simulink model "controller_observer_AllAgents_v5"  (Simulink 8.4)
settings: Solver=ode1  StartTime=0.0  StopTime=inf  FixedStep=60*1  RelTol=1e-3
values from neighbouring files (symbols this model uses):
  Cpw = 4180    [readepJSONout.m]
  m_max = 3    [DesignParameters.m]
  mc_MAX = 2    [DesignParameters.m]
  Tamb_additive_Sensor_fault_time = 60*60*24*(Tamb_day_ofthe_add_fault + Tamb_time_ofthe_add_fault)    (= 129600)    [faults_setting.m]
  Tamb_additive_Sensor_fault_value = 10    [faults_setting.m]
  Tamb_mult_Sensor_fault_time = 60*60*24*(Tamb_day_ofthe_mult_fault + Tamb_time_ofthe_mult_fault)    (= 129600)    [faults_setting.m]
  Tamb_mult_Sensor_fault_value = 1.1    [faults_setting.m]
  Ts = 3600/dat.Timestep.Timestep_0x20_1.number_of_timesteps_per_hour    [readepJSONout.m]
  Tst_C_additive_Sensor_fault_time = 60*60*24*(Tst_C_day_ofthe_add_fault + Tst_C_time_ofthe_add_fault)    (= 129600)    [faults_setting.m]
  Tst_C_additive_Sensor_fault_value = 10    [faults_setting.m]
  Tst_C_mult_Sensor_fault_time = 60*60*24*(Tst_C_day_ofthe_mult_fault + Tst_C_time_ofthe_mult_fault)    (= 129600)    [faults_setting.m]
  Tst_C_mult_Sensor_fault_value = 1.1    [faults_setting.m]
  Tst_H_additive_Sensor_fault_time = 60*60*24*(Tst_H_day_ofthe_add_fault + Tst_H_time_ofthe_add_fault)    (= 129600)    [faults_setting.m]
  Tst_H_additive_Sensor_fault_value = 10    [faults_setting.m]
  Tst_H_mult_Sensor_fault_time = 60*60*24*(Tst_H_day_ofthe_mult_fault + Tst_H_time_ofthe_mult_fault)    (= 129600)    [faults_setting.m]
  Tst_H_mult_Sensor_fault_value = 1.1    [faults_setting.m]
undefined symbols (not defined in the file or in its folder): building, COP, Ehp_max, fsa_E, fsa_ET, fsa_N, fsa_NT, fsa_W, fsa_WT, h_E, h_ET, h_N, h_NT, h_W, h_WT, K_NtoNT, K_NTtoN, Ke_E, Ke_ET, Ke_N, Ke_NT, Ke_WT, Km_E, Km_ET, Km_N, Km_NT, Km_W, Km_WT, Kout_N, Kout_NT, Kout_W, Kout_WT, Kst, Ksta, Kstcij, KstRef, Kw_E, Kw_ET, Kw_N, Kw_NT, Kw_W, Kw_WT
libraries: simulink x61
(part 36 of 36)

== system controller_observer_AllAgents_v5/Controller/MultiClass1Pod3_Agent/Threshold_hcoil/counter ==
  1 : Constant  SampleTime=Ts
  Delay2 : Delay  DelayLength=1  InitialCondition=0  SampleTime=Ts
  Sum : Sum  Inputs=|++
  Out1 : Outport
  Out2 : Outport  Port=2
  wires:
  1/1 -> Sum/2
  Delay2/1 -> Out1/1, Sum/1
  Sum/1 -> Out2/1, Delay2/1

== system controller_observer_AllAgents_v5/Controller/MultiClass1Pod3_Agent/Threshold_sa/If Action Subsystem2 ==
  In1 : Inport
  Action Port : ActionPort
  Out1 : Outport
  wires:
  In1/1 -> Out1/1

== system controller_observer_AllAgents_v5/Controller/MultiClass1Pod3_Agent/Threshold_sa/If Action Subsystem3 ==
  In1 : Inport
  Action Port : ActionPort
  Out1 : Outport
  wires:
  In1/1 -> Out1/1

== system controller_observer_AllAgents_v5/Controller/MultiClass1Pod3_Agent/Threshold_sa/counter ==
  1 : Constant  SampleTime=Ts
  Delay2 : Delay  DelayLength=1  InitialCondition=0  SampleTime=Ts
  Sum : Sum  Inputs=|++
  Out1 : Outport
  Out2 : Outport  Port=2
  wires:
  1/1 -> Sum/2
  Delay2/1 -> Out1/1, Sum/1
  Sum/1 -> Out2/1, Delay2/1

== system controller_observer_AllAgents_v5/Controller/MultiClass1Pod3_Agent/Threshold_zone/If Action Subsystem2 ==
  In1 : Inport
  Action Port : ActionPort
  Out1 : Outport
  wires:
  In1/1 -> Out1/1

== system controller_observer_AllAgents_v5/Controller/MultiClass1Pod3_Agent/Threshold_zone/If Action Subsystem3 ==
  In1 : Inport
  Action Port : ActionPort
  Out1 : Outport
  wires:
  In1/1 -> Out1/1

== system controller_observer_AllAgents_v5/Controller/MultiClass1Pod3_Agent/Threshold_zone/counter ==
  1 : Constant  SampleTime=Ts
  Delay2 : Delay  DelayLength=1  InitialCondition=0  SampleTime=Ts
  Sum : Sum  Inputs=|++
  Out1 : Outport
  Out2 : Outport  Port=2
  wires:
  1/1 -> Sum/2
  Delay2/1 -> Out1/1, Sum/1
  Sum/1 -> Out2/1, Delay2/1

== system controller_observer_AllAgents_v5/Controller/MultiClass2Pod1_Agent/Threshold_coil/If Action Subsystem2 ==
  In1 : Inport
  Action Port : ActionPort
  Out1 : Outport
  wires:
  In1/1 -> Out1/1

== system controller_observer_AllAgents_v5/Controller/MultiClass2Pod1_Agent/Threshold_coil/If Action Subsystem3 ==
  In1 : Inport
  Action Port : ActionPort
  Out1 : Outport
  wires:
  In1/1 -> Out1/1

== system controller_observer_AllAgents_v5/Controller/MultiClass2Pod1_Agent/Threshold_coil/counter ==
  1 : Constant  SampleTime=Ts
  Delay2 : Delay  DelayLength=1  InitialCondition=0  SampleTime=Ts
  Sum : Sum  Inputs=|++
  Out1 : Outport
  Out2 : Outport  Port=2
  wires:
  1/1 -> Sum/2
  Delay2/1 -> Out1/1, Sum/1
  Sum/1 -> Out2/1, Delay2/1

== system controller_observer_AllAgents_v5/Controller/MultiClass2Pod1_Agent/Threshold_hcoil/If Action Subsystem2 ==
  In1 : Inport
  Action Port : ActionPort
  Out1 : Outport
  wires:
  In1/1 -> Out1/1

== system controller_observer_AllAgents_v5/Controller/MultiClass2Pod1_Agent/Threshold_hcoil/If Action Subsystem3 ==
  In1 : Inport
  Action Port : ActionPort
  Out1 : Outport
  wires:
  In1/1 -> Out1/1

== system controller_observer_AllAgents_v5/Controller/MultiClass2Pod1_Agent/Threshold_hcoil/counter ==
  1 : Constant  SampleTime=Ts
  Delay2 : Delay  DelayLength=1  InitialCondition=0  SampleTime=Ts
  Sum : Sum  Inputs=|++
  Out1 : Outport
  Out2 : Outport  Port=2
  wires:
  1/1 -> Sum/2
  Delay2/1 -> Out1/1, Sum/1
  Sum/1 -> Out2/1, Delay2/1

== system controller_observer_AllAgents_v5/Controller/MultiClass2Pod1_Agent/Threshold_sa/If Action Subsystem2 ==
  In1 : Inport
  Action Port : ActionPort
  Out1 : Outport
  wires:
  In1/1 -> Out1/1

== system controller_observer_AllAgents_v5/Controller/MultiClass2Pod1_Agent/Threshold_sa/If Action Subsystem3 ==
  In1 : Inport
  Action Port : ActionPort
  Out1 : Outport
  wires:
  In1/1 -> Out1/1

== system controller_observer_AllAgents_v5/Controller/MultiClass2Pod1_Agent/Threshold_sa/counter ==
  1 : Constant  SampleTime=Ts
  Delay2 : Delay  DelayLength=1  InitialCondition=0  SampleTime=Ts
  Sum : Sum  Inputs=|++
  Out1 : Outport
  Out2 : Outport  Port=2
  wires:
  1/1 -> Sum/2
  Delay2/1 -> Out1/1, Sum/1
  Sum/1 -> Out2/1, Delay2/1

== system controller_observer_AllAgents_v5/Controller/MultiClass2Pod1_Agent/Threshold_zone/If Action Subsystem2 ==
  In1 : Inport
  Action Port : ActionPort
  Out1 : Outport
  wires:
  In1/1 -> Out1/1

== system controller_observer_AllAgents_v5/Controller/MultiClass2Pod1_Agent/Threshold_zone/If Action Subsystem3 ==
  In1 : Inport
  Action Port : ActionPort
  Out1 : Outport
  wires:
  In1/1 -> Out1/1

== system controller_observer_AllAgents_v5/Controller/MultiClass2Pod1_Agent/Threshold_zone/counter ==
  1 : Constant  SampleTime=Ts
  Delay2 : Delay  DelayLength=1  InitialCondition=0  SampleTime=Ts
  Sum : Sum  Inputs=|++
  Out1 : Outport
  Out2 : Outport  Port=2
  wires:
  1/1 -> Sum/2
  Delay2/1 -> Out1/1, Sum/1
  Sum/1 -> Out2/1, Delay2/1

== system controller_observer_AllAgents_v5/Controller/MultiClass2Pod2_Agent/Threshold_coil/If Action Subsystem2 ==
  In1 : Inport
  Action Port : ActionPort
  Out1 : Outport
  wires:
  In1/1 -> Out1/1

== system controller_observer_AllAgents_v5/Controller/MultiClass2Pod2_Agent/Threshold_coil/If Action Subsystem3 ==
  In1 : Inport
  Action Port : ActionPort
  Out1 : Outport
  wires:
  In1/1 -> Out1/1

== system controller_observer_AllAgents_v5/Controller/MultiClass2Pod2_Agent/Threshold_coil/counter ==
  1 : Constant  SampleTime=Ts
  Delay2 : Delay  DelayLength=1  InitialCondition=0  SampleTime=Ts
  Sum : Sum  Inputs=|++
  Out1 : Outport
  Out2 : Outport  Port=2
  wires:
  1/1 -> Sum/2
  Delay2/1 -> Out1/1, Sum/1
  Sum/1 -> Out2/1, Delay2/1

== system controller_observer_AllAgents_v5/Controller/MultiClass2Pod2_Agent/Threshold_hcoil/If Action Subsystem2 ==
  In1 : Inport
  Action Port : ActionPort
  Out1 : Outport
  wires:
  In1/1 -> Out1/1

== system controller_observer_AllAgents_v5/Controller/MultiClass2Pod2_Agent/Threshold_hcoil/If Action Subsystem3 ==
  In1 : Inport
  Action Port : ActionPort
  Out1 : Outport
  wires:
  In1/1 -> Out1/1

== system controller_observer_AllAgents_v5/Controller/MultiClass2Pod2_Agent/Threshold_hcoil/counter ==
  1 : Constant  SampleTime=Ts
  Delay2 : Delay  DelayLength=1  InitialCondition=0  SampleTime=Ts
  Sum : Sum  Inputs=|++
  Out1 : Outport
  Out2 : Outport  Port=2
  wires:
  1/1 -> Sum/2
  Delay2/1 -> Out1/1, Sum/1
  Sum/1 -> Out2/1, Delay2/1

== system controller_observer_AllAgents_v5/Controller/MultiClass2Pod2_Agent/Threshold_sa/If Action Subsystem2 ==
  In1 : Inport
  Action Port : ActionPort
  Out1 : Outport
  wires:
  In1/1 -> Out1/1

== system controller_observer_AllAgents_v5/Controller/MultiClass2Pod2_Agent/Threshold_sa/If Action Subsystem3 ==
  In1 : Inport
  Action Port : ActionPort
  Out1 : Outport
  wires:
  In1/1 -> Out1/1

== system controller_observer_AllAgents_v5/Controller/MultiClass2Pod2_Agent/Threshold_sa/counter ==
  1 : Constant  SampleTime=Ts
  Delay2 : Delay  DelayLength=1  InitialCondition=0  SampleTime=Ts
  Sum : Sum  Inputs=|++
  Out1 : Outport
  Out2 : Outport  Port=2
  wires:
  1/1 -> Sum/2
  Delay2/1 -> Out1/1, Sum/1
  Sum/1 -> Out2/1, Delay2/1

== system controller_observer_AllAgents_v5/Controller/MultiClass2Pod2_Agent/Threshold_zone/If Action Subsystem2 ==
  In1 : Inport
  Action Port : ActionPort
  Out1 : Outport
  wires:
  In1/1 -> Out1/1

== system controller_observer_AllAgents_v5/Controller/MultiClass2Pod2_Agent/Threshold_zone/If Action Subsystem3 ==
  In1 : Inport
  Action Port : ActionPort
  Out1 : Outport
  wires:
  In1/1 -> Out1/1

== system controller_observer_AllAgents_v5/Controller/MultiClass2Pod2_Agent/Threshold_zone/counter ==
  1 : Constant  SampleTime=Ts
  Delay2 : Delay  DelayLength=1  InitialCondition=0  SampleTime=Ts
  Sum : Sum  Inputs=|++
  Out1 : Outport
  Out2 : Outport  Port=2
  wires:
  1/1 -> Sum/2
  Delay2/1 -> Out1/1, Sum/1
  Sum/1 -> Out2/1, Delay2/1

== system controller_observer_AllAgents_v5/Controller/MultiClass2Pod3_Agent/Threshold_coil/If Action Subsystem2 ==
  In1 : Inport
  Action Port : ActionPort
  Out1 : Outport
  wires:
  In1/1 -> Out1/1

== system controller_observer_AllAgents_v5/Controller/MultiClass2Pod3_Agent/Threshold_coil/If Action Subsystem3 ==
  In1 : Inport
  Action Port : ActionPort
  Out1 : Outport
  wires:
  In1/1 -> Out1/1

== system controller_observer_AllAgents_v5/Controller/MultiClass2Pod3_Agent/Threshold_coil/counter ==
  1 : Constant  SampleTime=Ts
  Delay2 : Delay  DelayLength=1  InitialCondition=0  SampleTime=Ts
  Sum : Sum  Inputs=|++
  Out1 : Outport
  Out2 : Outport  Port=2
  wires:
  1/1 -> Sum/2
  Delay2/1 -> Out1/1, Sum/1
  Sum/1 -> Out2/1, Delay2/1

== system controller_observer_AllAgents_v5/Controller/MultiClass2Pod3_Agent/Threshold_hcoil/If Action Subsystem2 ==
  In1 : Inport
  Action Port : ActionPort
  Out1 : Outport
  wires:
  In1/1 -> Out1/1

== system controller_observer_AllAgents_v5/Controller/MultiClass2Pod3_Agent/Threshold_hcoil/If Action Subsystem3 ==
  In1 : Inport
  Action Port : ActionPort
  Out1 : Outport
  wires:
  In1/1 -> Out1/1

== system controller_observer_AllAgents_v5/Controller/MultiClass2Pod3_Agent/Threshold_hcoil/counter ==
  1 : Constant  SampleTime=Ts
  Delay2 : Delay  DelayLength=1  InitialCondition=0  SampleTime=Ts
  Sum : Sum  Inputs=|++
  Out1 : Outport
  Out2 : Outport  Port=2
  wires:
  1/1 -> Sum/2
  Delay2/1 -> Out1/1, Sum/1
  Sum/1 -> Out2/1, Delay2/1

== system controller_observer_AllAgents_v5/Controller/MultiClass2Pod3_Agent/Threshold_sa/If Action Subsystem2 ==
  In1 : Inport
  Action Port : ActionPort
  Out1 : Outport
  wires:
  In1/1 -> Out1/1

== system controller_observer_AllAgents_v5/Controller/MultiClass2Pod3_Agent/Threshold_sa/If Action Subsystem3 ==
  In1 : Inport
  Action Port : ActionPort
  Out1 : Outport
  wires:
  In1/1 -> Out1/1

== system controller_observer_AllAgents_v5/Controller/MultiClass2Pod3_Agent/Threshold_sa/counter ==
  1 : Constant  SampleTime=Ts
  Delay2 : Delay  DelayLength=1  InitialCondition=0  SampleTime=Ts
  Sum : Sum  Inputs=|++
  Out1 : Outport
  Out2 : Outport  Port=2
  wires:
  1/1 -> Sum/2
  Delay2/1 -> Out1/1, Sum/1
  Sum/1 -> Out2/1, Delay2/1

== system controller_observer_AllAgents_v5/Controller/MultiClass2Pod3_Agent/Threshold_zone/If Action Subsystem2 ==
  In1 : Inport
  Action Port : ActionPort
  Out1 : Outport
  wires:
  In1/1 -> Out1/1

== system controller_observer_AllAgents_v5/Controller/MultiClass2Pod3_Agent/Threshold_zone/If Action Subsystem3 ==
  In1 : Inport
  Action Port : ActionPort
  Out1 : Outport
  wires:
  In1/1 -> Out1/1

== system controller_observer_AllAgents_v5/Controller/MultiClass2Pod3_Agent/Threshold_zone/counter ==
  1 : Constant  SampleTime=Ts
  Delay2 : Delay  DelayLength=1  InitialCondition=0  SampleTime=Ts
  Sum : Sum  Inputs=|++
  Out1 : Outport
  Out2 : Outport  Port=2
  wires:
  1/1 -> Sum/2
  Delay2/1 -> Out1/1, Sum/1
  Sum/1 -> Out2/1, Delay2/1

== system controller_observer_AllAgents_v5/Controller/Offices_Agent/Threshold_coil/If Action Subsystem2 ==
  In1 : Inport
  Action Port : ActionPort
  Out1 : Outport
  wires:
  In1/1 -> Out1/1

== system controller_observer_AllAgents_v5/Controller/Offices_Agent/Threshold_coil/If Action Subsystem3 ==
  In1 : Inport
  Action Port : ActionPort
  Out1 : Outport
  wires:
  In1/1 -> Out1/1

== system controller_observer_AllAgents_v5/Controller/Offices_Agent/Threshold_coil/counter ==
  1 : Constant  SampleTime=Ts
  Delay2 : Delay  DelayLength=1  InitialCondition=0  SampleTime=Ts
  Sum : Sum  Inputs=|++
  Out1 : Outport
  Out2 : Outport  Port=2
  wires:
  1/1 -> Sum/2
  Delay2/1 -> Out1/1, Sum/1
  Sum/1 -> Out2/1, Delay2/1

== system controller_observer_AllAgents_v5/Controller/Offices_Agent/Threshold_hcoil/If Action Subsystem2 ==
  In1 : Inport
  Action Port : ActionPort
  Out1 : Outport
  wires:
  In1/1 -> Out1/1

== system controller_observer_AllAgents_v5/Controller/Offices_Agent/Threshold_hcoil/If Action Subsystem3 ==
  In1 : Inport
  Action Port : ActionPort
  Out1 : Outport
  wires:
  In1/1 -> Out1/1

== system controller_observer_AllAgents_v5/Controller/Offices_Agent/Threshold_hcoil/counter ==
  1 : Constant  SampleTime=Ts
  Delay2 : Delay  DelayLength=1  InitialCondition=0  SampleTime=Ts
  Sum : Sum  Inputs=|++
  Out1 : Outport
  Out2 : Outport  Port=2
  wires:
  1/1 -> Sum/2
  Delay2/1 -> Out1/1, Sum/1
  Sum/1 -> Out2/1, Delay2/1

== system controller_observer_AllAgents_v5/Controller/Offices_Agent/Threshold_sa/If Action Subsystem2 ==
  In1 : Inport
  Action Port : ActionPort
  Out1 : Outport
  wires:
  In1/1 -> Out1/1

== system controller_observer_AllAgents_v5/Controller/Offices_Agent/Threshold_sa/If Action Subsystem3 ==
  In1 : Inport
  Action Port : ActionPort
  Out1 : Outport
  wires:
  In1/1 -> Out1/1

== system controller_observer_AllAgents_v5/Controller/Offices_Agent/Threshold_sa/counter ==
  1 : Constant  SampleTime=Ts
  Delay2 : Delay  DelayLength=1  InitialCondition=0  SampleTime=Ts
  Sum : Sum  Inputs=|++
  Out1 : Outport
  Out2 : Outport  Port=2
  wires:
  1/1 -> Sum/2
  Delay2/1 -> Out1/1, Sum/1
  Sum/1 -> Out2/1, Delay2/1

== system controller_observer_AllAgents_v5/Controller/Offices_Agent/Threshold_zone/If Action Subsystem2 ==
  In1 : Inport
  Action Port : ActionPort
  Out1 : Outport
  wires:
  In1/1 -> Out1/1

== system controller_observer_AllAgents_v5/Controller/Offices_Agent/Threshold_zone/If Action Subsystem3 ==
  In1 : Inport
  Action Port : ActionPort
  Out1 : Outport
  wires:
  In1/1 -> Out1/1

== system controller_observer_AllAgents_v5/Controller/Offices_Agent/Threshold_zone/counter ==
  1 : Constant  SampleTime=Ts
  Delay2 : Delay  DelayLength=1  InitialCondition=0  SampleTime=Ts
  Sum : Sum  Inputs=|++
  Out1 : Outport
  Out2 : Outport  Port=2
  wires:
  1/1 -> Sum/2
  Delay2/1 -> Out1/1, Sum/1
  Sum/1 -> Out2/1, Delay2/1
